simulink model "CDF_VSVPWM_Grid_Parallel"  (R2024b)
settings: Solver=VariableStepAuto  StartTime=0.0  StopTime=0.1  FixedStep=1e-7  MaxStep=1e-5  RelTol=1e-4
InitFcn:
  | Ts=1/10e3;
  | Udc=600;
  | C=4000e-6;
  | m=0.7;
libraries: simulink x24, spsMosfetLib x24, spsSeriesRLCBranchLib x16, spsDiodeLib x12, spsabctoAlphaBetaZeroLib x5, spsThreePhaseVIMeasurementLib x4, spsVoltageMeasurementLib x4, spsAlphaBetaZerotoabcLib x2, spsStairGeneratorLib x2, spsOnDelayLib x2, spsdq0toAlphaBetaZeroLib x2, spsBreakerLib x1
(part 1 of 5)
settings Model: SimulationMode=accelerator  PauseTimes=0  Creator=chenl  LastModifiedBy=ZKY
settings SolverCC: AlgebraicLoopSolver=TrustRegion
settings DataIOCC: ReturnWorkspaceOutputs=off
settings DebuggingCC: ArithmeticOperatorsInVariantConditions=warning
settings SFSimCC: CustomCodeUndefinedFunction=UseInterfaceOnly
settings RTWCC: GPUComputeCapability=3.5

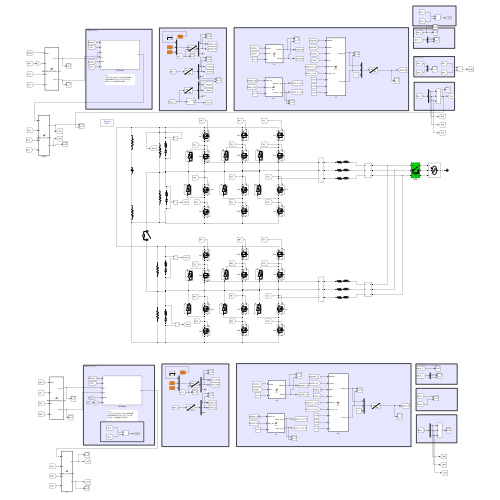
[thumbnail: Simulink's stored preview of the top-level diagram]
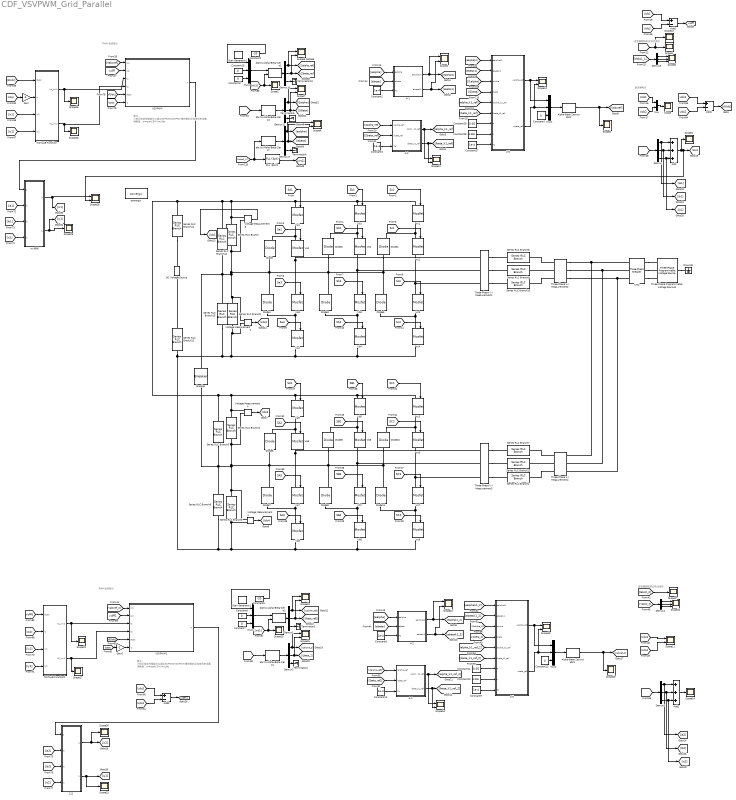
== system CDF_VSVPWM_Grid_Parallel ==
  note: PWM发波部分
  note: 备注：
      | 三相正弦信号幅值可以通过SVPWM/VSVPWM模块里的正弦信号来设置
      | 调制度：m=sqrt(3)*Vm/Udc
  note: 直流侧电压
  note: 逆变器侧线电压电流波形
  note: PWM发波部分
  note: 备注：
      | 三相正弦信号幅值可以通过SVPWM/VSVPWM模块里的正弦信号来设置
      | 调制度：m=sqrt(3)*Vm/Udc
  note: 逆变器侧线电压电流波形
  111 : MATLAB Function  TreatAsAtomicUnit=on
  4-1 : MATLAB Function  TreatAsAtomicUnit=on
  4-2 : MATLAB Function  TreatAsAtomicUnit=on
  4-3 : MATLAB Function  TreatAsAtomicUnit=on
  4-4 : MATLAB Function  TreatAsAtomicUnit=on
  4-5 : MATLAB Function  TreatAsAtomicUnit=on
  4-8 : MATLAB Function  TreatAsAtomicUnit=on
  Add : Sum  Inputs=+++
  Add1 : Sum  Inputs=+-
  Add2 : Sum  Inputs=+++
  Add3 : Sum  Inputs=+-
  Add4 : Sum  Inputs=+-
  Alpha-Beta-Zero to abc1 : spsAlphaBetaZerotoabcLib/Alpha-Beta-Zero to abc
      settings: LibrarySourceBlock=|
      | sps_lib/Control/Alpha-Beta-Zero
      | to abc
  Alpha-Beta-Zero to abc2 : spsAlphaBetaZerotoabcLib/Alpha-Beta-Zero to abc
      settings: LibrarySourceBlock=|
      | sps_lib/Control/Alpha-Beta-Zero
      | to abc
  Breaker : spsBreakerLib/Breaker  InitialState=0  SwitchingTimes=0  External=off  BreakerResistance=0.001  SnubberResistance=1e8  SnubberCapacitance=inf  Measurements=None  MoreParameters=on  NoBreakLoop=off
  Constant1 : Constant  Value=-20
  Constant10 : Constant  Value=0.005
  Constant11 : Constant  Value=0.05
  Constant{2..3,8..9,12,27} (6 alike) : Constant  Value=1e-4
  Constant{4,6..7,13,15,17} (6 alike) : Constant  Value=0
  Constant28 : Constant  Value=0.005
  Constant29 : Constant  Value=0.05
  Constant5 : Constant  Value=-20
  DC Voltage Source : spsDCVoltageSourceLib/DC Voltage Source  Amplitude=Udc  Measurements=None
  Demux{,1..5,10,12,17} (9 alike) : Demux  Outputs=3
  Diode{,1..11} (12 alike) : spsDiodeLib/Diode  Ron=0.001  Lon=0  Vf=0.8  IC=0  UseSnubber=0  Rs=500  Cs=250e-9  Measurements=on
  Gain : Gain  Gain=-1
  Gain2 : Gain  Gain=-1
  Ground3 : spsGroundLib/Ground  LConnTagsString=a
  KM4 : spsThreePhaseBreakerLib/Three-Phase Breaker  InitialState=open  SwitchA=on  SwitchB=on  SwitchC=on  SwitchTimes=0  External=off  BreakerResistance=0.001  SnubberResistance=1e6  SnubberCapacitance=inf  Measurements=None
  MATLAB Function : MATLAB Function  TreatAsAtomicUnit=on
  MATLAB Function1 : MATLAB Function  TreatAsAtomicUnit=on
  Mux : Mux  Inputs=2
  Mux{1..4} (4 alike) : Mux  Inputs=3
  PLL (3ph)2 : spsPLL3phLib/PLL (3ph)  Fmin=45  Par_Init=[0, 50]  ParK=[180, 3200, 0]  TcD=1e-4  MaxRateChangeFreq=12  FilterCutOffFreq=25  Ts=0  AGC=on
  Scope{,1..3,8,10,14..17,20..21,26..27,29..31} (17 alike) : Scope
  Scope{4..5,7,9,11..13,18..19,22..23,25,28} (13 alike) : Scope  NumInputPorts=2
  Scope24 : Scope  NumInputPorts=3
  Scope6 : Scope  NumInputPorts=3
  Series RLC Branch : spsSeriesRLCBranchLib/Series RLC Branch  LConnTagsString=__new0  RConnTagsString=__new0  BranchType=RC  Resistance=1  Inductance=1e-3  SetiL0=off  InitialCurrent=0  Capacitance=860e-6  Setx0=on  InitialVoltage=280  Measurements=None
  Series RLC Branch1 : spsSeriesRLCBranchLib/Series RLC Branch  LConnTagsString=__new0  RConnTagsString=__new0  BranchType=RC  Resistance=0.001  Inductance=1e-3  SetiL0=off  InitialCurrent=0  Capacitance=860e-6  Setx0=on  InitialVoltage=320  Measurements=None
  Series RLC Branch10 : spsSeriesRLCBranchLib/Series RLC Branch  LConnTagsString=__new0  RConnTagsString=__new0  BranchType=RL  Resistance=0.05  Inductance=3e-3  SetiL0=off  InitialCurrent=0  Capacitance=1e-6  Setx0=off  InitialVoltage=0  Measurements=None
  Series RLC Branch11 : spsSeriesRLCBranchLib/Series RLC Branch  LConnTagsString=__new0  RConnTagsString=__new0  BranchType=R  Resistance=0.00001  Inductance=1e-3  SetiL0=off  InitialCurrent=0  Capacitance=1e-6  Setx0=off  InitialVoltage=0  Measurements=None
  Series RLC Branch12 : spsSeriesRLCBranchLib/Series RLC Branch  LConnTagsString=__new0  RConnTagsString=__new0  BranchType=R  Resistance=0.00001  Inductance=1e-3  SetiL0=off  InitialCurrent=0  Capacitance=1e-6  Setx0=off  InitialVoltage=0  Measurements=None
  Series RLC Branch13 : spsSeriesRLCBranchLib/Series RLC Branch  LConnTagsString=__new0  RConnTagsString=__new0  BranchType=RL  Resistance=0.05  Inductance=5e-3  SetiL0=off  InitialCurrent=0  Capacitance=1e-6  Setx0=off  InitialVoltage=0  Measurements=None
  Series RLC Branch{5..6,14..15} (4 alike) : spsSeriesRLCBranchLib/Series RLC Branch  LConnTagsString=__new0  RConnTagsString=__new0  BranchType=R  Resistance=1e4  Inductance=1e-3  SetiL0=off  InitialCurrent=0  Capacitance=C  Setx0=on  InitialVoltage=Udc/2  Measurements=None
  Series RLC Branch2 : spsSeriesRLCBranchLib/Series RLC Branch  LConnTagsString=__new0  RConnTagsString=__new0  BranchType=RC  Resistance=0.001  Inductance=1e-3  SetiL0=off  InitialCurrent=0  Capacitance=860e-6  Setx0=off  InitialVoltage=300  Measurements=None
  Series RLC Branch3 : spsSeriesRLCBranchLib/Series RLC Branch  LConnTagsString=__new0  RConnTagsString=__new0  BranchType=RC  Resistance=0.001  Inductance=1e-3  SetiL0=off  InitialCurrent=0  Capacitance=860e-6  Setx0=off  InitialVoltage=300  Measurements=None
  Series RLC Branch4 : spsSeriesRLCBranchLib/Series RLC Branch  LConnTagsString=__new0  RConnTagsString=__new0  BranchType=RL  Resistance=0.05  Inductance=5e-3  SetiL0=off  InitialCurrent=0  Capacitance=1e-6  Setx0=off  InitialVoltage=0  Measurements=None
  Series RLC Branch7 : spsSeriesRLCBranchLib/Series RLC Branch  LConnTagsString=__new0  RConnTagsString=__new0  BranchType=RL  Resistance=0.05  Inductance=5e-3  SetiL0=off  InitialCurrent=0  Capacitance=1e-6  Setx0=off  InitialVoltage=0  Measurements=None
  Series RLC Branch8 : spsSeriesRLCBranchLib/Series RLC Branch  LConnTagsString=__new0  RConnTagsString=__new0  BranchType=RL  Resistance=0.05  Inductance=3e-3  SetiL0=off  InitialCurrent=0  Capacitance=1e-6  Setx0=off  InitialVoltage=0  Measurements=None
  Series RLC Branch9 : spsSeriesRLCBranchLib/Series RLC Branch  LConnTagsString=__new0  RConnTagsString=__new0  BranchType=RL  Resistance=0.05  Inductance=3e-3  SetiL0=off  InitialCurrent=0  Capacitance=1e-6  Setx0=off  InitialVoltage=0  Measurements=None
  Stair Generator1 : spsStairGeneratorLib/Stair Generator  t=[0, 0.1, 0.2]  e=[-10,-5, -10]  Ts=0
  Stair Generator2 : spsStairGeneratorLib/Stair Generator  t=[0, 0.1, 0.2]  e=[-10,-5, -10]  Ts=0
  Terminator{1..2,6,8,10} (5 alike) : Terminator
  Three-Phase Programmable Voltage Source1 : spsThreePhaseProgrammableVoltageSourceLib/Three-Phase Programmable Voltage Source  PositiveSequence="[380  0 50]"  VariationEntity=Amplitude  VariationType=Table of time-amplitude pairs  VariationTypeAlt=Step  VariationStep=0.5  VariationRate=10  VariationMagnitude=0.3  VariationFrequency=2  VariationTiming=[1 2]  VariationPhaseA=on  Amplitudes=[1.0]  TimeValues=[0]  HarmonicGeneration=off  HarmonicA=[3 0.064 0 0]  HarmonicB=[5 0.048 0 2]  Timing="[0.05  0.15]"  BusType=swing  Pref=0  Qref=0  Qmin=-inf  Qmax=inf
  Three-Phase V-I Measurement1 : spsThreePhaseVIMeasurementLib/Three-Phase V-I Measurement  VoltageMeasurement=phase-to-ground  SetLabelV=on  LabelV=Vabc1_1  Vpu=off  VpuLL=off  CurrentMeasurement=yes  SetLabelI=on  LabelI=Iabc1_1  Ipu=off  Pbase=100e6  Vbase=500e3  OutputType=Complex  PhasorSimulation=off  PSBequivalent=0
  Three-Phase V-I Measurement2 : spsThreePhaseVIMeasurementLib/Three-Phase V-I Measurement  VoltageMeasurement=phase-to-ground  SetLabelV=on  LabelV=Vabc2_1  Vpu=off  VpuLL=off  CurrentMeasurement=no  SetLabelI=on  LabelI=Iabc2  Ipu=off  Pbase=100e6  Vbase=500e3  OutputType=Complex  PhasorSimulation=off  PSBequivalent=0
  Three-Phase V-I Measurement3 : spsThreePhaseVIMeasurementLib/Three-Phase V-I Measurement  VoltageMeasurement=phase-to-ground  SetLabelV=on  LabelV=Vabc1_2  Vpu=off  VpuLL=off  CurrentMeasurement=yes  SetLabelI=on  LabelI=Iabc1_2  Ipu=off  Pbase=100e6  Vbase=500e3  OutputType=Complex  PhasorSimulation=off  PSBequivalent=0
  Three-Phase V-I Measurement4 : spsThreePhaseVIMeasurementLib/Three-Phase V-I Measurement  VoltageMeasurement=phase-to-ground  SetLabelV=on  LabelV=Vabc2_2  Vpu=off  VpuLL=off  CurrentMeasurement=yes  SetLabelI=on  LabelI=Iabc2_2  Ipu=off  Pbase=100e6  Vbase=500e3  OutputType=Complex  PhasorSimulation=off  PSBequivalent=0
  VSVPWM : Subsystem
  VSVPWM1 : Subsystem
  Va{1..8} (8 alike) : spsMosfetLib/Mosfet  Ron=0.1  Lon=0  Rd=0.01  Vfd=0  IC=0  Rs=1e5  Cs=inf  Measurements=on
  Vb{1..8} (8 alike) : spsMosfetLib/Mosfet  Ron=0.1  Lon=0  Rd=0.01  Vfd=0  IC=0  Rs=1e5  Cs=inf  Measurements=on
  Vc{1..8} (8 alike) : spsMosfetLib/Mosfet  Ron=0.1  Lon=0  Rd=0.01  Vfd=0  IC=0  Rs=1e5  Cs=inf  Measurements=on
  Voltage Measurement{1..2,4,8} (4 alike) : spsVoltageMeasurementLib/Voltage Measurement  PhasorSimulation=off  OutputType=Complex  PSBequivalent=0
  abc to Alpha-Beta-Zero1 : spsabctoAlphaBetaZeroLib/abc to Alpha-Beta-Zero
  abc to Alpha-Beta-Zero4 : spsabctoAlphaBetaZeroLib/abc to Alpha-Beta-Zero
  abc to Alpha-Beta-Zero7 : spsabctoAlphaBetaZeroLib/abc to Alpha-Beta-Zero
  dq0 to Alpha-Beta-Zero1 : spsdq0toAlphaBetaZeroLib/dq0 to Alpha-Beta-Zero  Alignment=90 degrees behind phase A axis
  dq0 to Alpha-Beta-Zero2 : spsdq0toAlphaBetaZeroLib/dq0 to Alpha-Beta-Zero  Alignment=90 degrees behind phase A axis
  ixiy映射 : MATLAB Function  TreatAsAtomicUnit=on
  powergui : spspowerguiLib/powergui  Priority=1  SimulationMode=Discrete  SampleTime=1e-6  frequency=60  Iterations=50  frequencyindice=0  Pbase=100e6  ErrMax=1e-4  UnitsV=kV  UnitsW=MW  buscounter=0  FunctionMessages=off  echomessages=off  EnableUseOfTLC=off  CurrentSourceSwitches=off  DisableSnubberDevices=off  DisableRonSwitches=off  DisableVfSwitches=off  DisplayEquations=off  AutomaticDiscreteSolvers=off  SolverType=Tustin/Backward Euler (TBE)  Interpol=off  ExternalGateDelay=off  methode=off  Ts=100  x0status=blocks  SPID=on  SwTol=0  HookPort=off  NonlinearTolerance=1e-4  nMaxIteration=100  ContinueOnMaxIteration=off  RestoreLinks=warning  ResistiveCurrentMeasurement=off  Frange=[0:2:500]  Ylog=off  Xlog=on  ShowGrid=off  save=off  variable=ZData  structure=0  ZoomFFT=off  StartTime=0.0  cycles=1  DisplayStyle=1  fundamental=60  FreqAxis=off  MaxFrequency=1000  frequencyindicesteady=1  RmsSteady=1  display=off
  tag scope: Udc1=global, Udc2=global, Udc3=global, Udc4=global, izscc=global, vidff1=global, vidff=global
  wires:
  [Sa1] -> Va1/1
  [Sb1] -> Vb1/1
  [Sc1] -> Vc1/1
  [Sa4] -> Va4/1
  [Sb4] -> Vb4/1
  [Sc4] -> Vc4/1
  [Iabc1_1] -> Scope10/1, Scope1/1
  Voltage Measurement2/1 -> [Udc2]
  [Vabc1_1] -> Demux5/1
  Demux5/1 -> Scope6/1
  Demux5/2 -> Scope6/2
  Demux5/3 -> Scope6/3
  Voltage Measurement8/1 -> [Udc1]
  [Sa2] -> Va2/1
  [Sb2] -> Vb2/1
  [Sc2] -> Vc2/1
  [Sa3] -> Va3/1
  [Sb3] -> Vb3/1
  [Sc3] -> Vc3/1
  Mux4/1 -> dq0 to Alpha-Beta-Zero1/1
  Demux10/1 -> Scope5/1, [i2alpha_ref]
  Demux10/3 -> Terminator10/1
  Constant17/1 -> Mux4/3
  Demux10/2 -> Scope5/2, [i2beta_ref]
  [wt1] -> Scope2/1, dq0 to Alpha-Beta-Zero1/2
  Constant15/1 -> Mux4/2
  dq0 to Alpha-Beta-Zero1/1 -> Demux10/1
  [Iabc1_1] -> abc to Alpha-Beta-Zero4/1
  Demux12/3 -> Terminator6/1
  Demux12/2 -> Scope7/2, [I1beta]
  Demux12/1 -> Scope7/1, [I1alpha]
  abc to Alpha-Beta-Zero4/1 -> Demux12/1
  Demux17/2 -> Scope9/2, [ebeta]
  Demux17/3 -> Terminator8/1
  Demux17/1 -> Scope9/1, [ealpha]
  [Vabc2_1] -> abc to Alpha-Beta-Zero7/1, PLL (3ph)2/1
  PLL (3ph)2/2 -> [wt1]
  abc to Alpha-Beta-Zero7/1 -> Demux17/1
  Constant2/1 -> 4-1/3(Ts)
  [ealpha] -> 4-1/1(ealpha)
  [ebeta] -> 4-1/2(ebeta)
  4-1/2(ebetak1) -> [ebetak1], Scope4/2
  4-1/1(ealphak1) -> Scope4/1, [ealphak1]
  [i2alpha_ref] -> 4-3/1(ialpha_ref)
  [i2beta_ref] -> 4-3/2(ibeta_ref)
  Constant3/1 -> 4-3/3(Ts)
  [ealphak1] -> 4-8/1(ealphak1)
  [ebetak1] -> 4-8/2(ebetak1)
  [I1alpha] -> 4-8/3(ialpha)
  [I1beta] -> 4-8/4(ibeta)
  4-3/1(ialpha_k1_ref) -> Scope12/1, [ialpha_k1_ref]
  4-3/2(ibeta_k1_ref) -> Scope12/2, [ibeta_k1_ref]
  [ialpha_k1_ref] -> 4-8/5(ialpha_k1_ref)
  [ibeta_k1_ref] -> 4-8/6(ibeta_k1_ref)
  Constant29/1 -> 4-8/7(R)
  Constant28/1 -> 4-8/8(L)
  Constant27/1 -> 4-8/9(Ts)
  Constant4/1 -> Mux1/3
  Alpha-Beta-Zero to abc2/1 -> Scope8/1, [Vabcref]
  4-8/1(ualpha_ref) -> Scope13/1, Mux1/1
  4-8/2(ubeta_ref) -> Scope13/2, Mux1/2
  Mux1/1 -> Alpha-Beta-Zero to abc2/1
  [Vabcref] -> VSVPWM/1(In1)
  Constant1/1 -> Mux4/1
  [SA1] -> Va5/1
  Voltage Measurement1/1 -> [Udc4]
  [SA3] -> Va7/1
  Voltage Measurement4/1 -> [Udc3]
  [SC2] -> Vc6/1
  [SB4] -> Vb8/1
  [SC1] -> Vc5/1
  [SB1] -> Vb5/1
  [SB2] -> Vb6/1
  [SB3] -> Vb7/1
  [SC4] -> Vc8/1
  [SA4] -> Va8/1
  [SA2] -> Va6/1
  [SC3] -> Vc7/1
  Alpha-Beta-Zero to abc1/1 -> Scope26/1, [Vabcref_2]
  [ealpha] -> 4-2/1(ealpha)
  [i2alpha_ref2] -> 4-4/1(ialpha_ref)
  Constant6/1 -> Mux3/2
  Demux4/1 -> Scope24/1
  [Iabc2_2] -> Scope17/1
  [ibeta_k1_ref_2] -> 4-5/6(ibeta_k1_ref)
  Mux2/1 -> Alpha-Beta-Zero to abc1/1
  [ialpha_k1_ref_2] -> 4-5/5(ialpha_k1_ref)
  Constant7/1 -> Mux3/3
  [Vabc2_2] -> Demux4/1
  Demux1/2 -> Scope23/2, [i2beta_ref2]
  Demux1/1 -> Scope23/1, [i2alpha_ref2]
  abc to Alpha-Beta-Zero1/1 -> Demux2/1
  dq0 to Alpha-Beta-Zero2/1 -> Demux1/1
  [ebetak1_2] -> 4-5/2(ebetak1)
  [Iabc1_2] -> abc to Alpha-Beta-Zero1/1
  [Vabcref_2] -> VSVPWM1/1(In1)
  [i2beta_ref2] -> 4-4/2(ibeta_ref)
  Constant5/1 -> Mux3/1
  4-2/1(ealphak1) -> Scope22/1, [ealphak1_2]
  Demux1/3 -> Terminator1/1
  Demux2/2 -> Scope25/2, [I1beta_2]
  [I1beta_2] -> 4-5/4(ibeta)
  Constant12/1 -> 4-4/3(Ts)
  Constant10/1 -> 4-5/8(L)
  Demux4/3 -> Scope24/3
  Mux3/1 -> dq0 to Alpha-Beta-Zero2/1
  Demux2/1 -> Scope25/1, [I1alpha_2]
  [ealphak1_2] -> 4-5/1(ealphak1)
  4-5/2(ubeta_ref) -> Scope19/2, Mux2/2
  [wt1] -> Scope20/1, dq0 to Alpha-Beta-Zero2/2
  4-2/2(ebetak1) -> [ebetak1_2], Scope22/2
  Constant8/1 -> 4-2/3(Ts)
  4-5/1(ualpha_ref) -> Scope19/1, Mux2/1
  Demux2/3 -> Terminator2/1
  Constant13/1 -> Mux2/3
  4-4/1(ialpha_k1_ref) -> Scope18/1, [ialpha_k1_ref_2]
  4-4/2(ibeta_k1_ref) -> Scope18/2, [ibeta_k1_ref_2]
  Demux4/2 -> Scope24/2
  [I1alpha_2] -> 4-5/3(ialpha)
  [ebeta] -> 4-2/2(ebeta)
  Constant9/1 -> 4-5/9(Ts)
  Constant11/1 -> 4-5/7(R)
  [Iabc1_1] -> Demux/1
  Demux/1 -> Add/1, [ia1]
  Demux/2 -> Add/2, [ib1]
  Demux/3 -> [ic1], Add/3
  Add/1 -> Scope3/1, Scope28/2, [izscc]
  [vidff] -> VSVPWM/2(In2)
  [Udc2] -> Add1/2
  [Udc1] -> Add1/1
  Add1/1 -> [vidff]
  [Udc3] -> Scope11/1
  [Udc4] -> Scope11/2
  Demux3/3 -> [ic2], Add2/3
  [Iabc1_1] -> Demux3/1
  Add2/1 -> Scope14/1
  Demux3/1 -> [ia2], Add2/1
  [vidff1] -> VSVPWM1/2(In2)
  Add3/1 -> [vidff1]
  [Udc4] -> Add3/2
  [Udc3] -> Add3/1
  [Udc1] -> Mux/1
  [Udc2] -> Mux/2
  Mux/1 -> Scope/1
  [Udc1] -> Add4/1
  [Udc2] -> Add4/2
  Add4/1 -> [dUdc]
  [dUdc] -> MATLAB Function/1(dUdc)
  [ix1] -> MATLAB Function/3(ix1)
  [iy1] -> MATLAB Function/4(iy1)
  MATLAB Function/1(k1_inv1) -> Scope15/1, VSVPWM/3(k1)
  MATLAB Function/2(k2_inv1) -> VSVPWM/4(k2), Scope16/1
  [izscc] -> Gain/1
  Gain/1 -> MATLAB Function/2(iz)
  [ix2] -> MATLAB Function1/3(ix1)
  [iy2] -> MATLAB Function1/4(iy1)
  [vidff1] -> MATLAB Function1/1(dUdc)
  Demux3/2 -> [ib2], Add2/2
  MATLAB Function1/1(k1_inv1) -> Scope21/1, VSVPWM1/3(k1)
  MATLAB Function1/2(k2_inv1) -> Scope27/1, VSVPWM1/4(k2)
  VSVPWM/1(N) -> ixiy映射/1(N)
  [ia1] -> ixiy映射/2(ia)
  [ic1] -> ixiy映射/4(ic)
  [ib1] -> ixiy映射/3(ib)
  ixiy映射/1(ix) -> [ix1], Scope28/1
  ixiy映射/2(iy) -> [iy1], Scope29/1
  111/2(iy) -> [iy2], Scope31/1
  VSVPWM1/1(N) -> 111/1(N)
  [ia2] -> 111/2(ia)
  111/1(ix) -> [ix2], Scope30/1
  [ic2] -> 111/4(ic)
  [ib2] -> 111/3(ib)
  [dUdc] -> VSVPWM/5(dUdc)
  [izscc] -> VSVPWM/6(iz)
  [dUdc] -> VSVPWM1/5(dUdc)
  Gain2/1 -> VSVPWM1/6(iz)
  [izscc] -> Gain2/1
  [izscc] -> MATLAB Function1/2(iz)
  connections:
  Series RLC Branch3/RConn1 -- Vb8/RConn1 -- Vc8/RConn1 -- Va8/RConn1 -- Series RLC Branch15/RConn1 -- Series RLC Branch1/RConn1 -- Voltage Measurement2/LConn2 -- Vc4/RConn1 -- Vb4/RConn1 -- Va4/RConn1 -- Series RLC Branch12/RConn1 -- Series RLC Branch6/RConn1 -- Voltage Measurement1/LConn2
  Three-Phase V-I Measurement1/RConn3 -- Series RLC Branch10/LConn1
  Three-Phase V-I Measurement1/RConn2 -- Series RLC Branch9/LConn1
  Three-Phase V-I Measurement1/RConn1 -- Series RLC Branch8/LConn1
  Series RLC Branch11/LConn1 -- DC Voltage Source/RConn1
  Series RLC Branch12/LConn1 -- DC Voltage Source/LConn1
  Va2/LConn1 -- Va1/RConn1 -- Diode/RConn1
  Vb2/LConn1 -- Diode2/RConn1 -- Vb1/RConn1
  Vc2/LConn1 -- Diode4/RConn1 -- Vc1/RConn1
  Va2/RConn1 -- Va3/LConn1 -- Three-Phase V-I Measurement1/LConn1
  Va3/RConn1 -- Diode1/LConn1 -- Va4/LConn1
  Vb3/RConn1 -- Diode3/LConn1 -- Vb4/LConn1
  Vb3/LConn1 -- Vb2/RConn1 -- Three-Phase V-I Measurement1/LConn2
  Vc3/RConn1 -- Vc4/LConn1 -- Diode5/LConn1
  Vc3/LConn1 -- Three-Phase V-I Measurement1/LConn3 -- Vc2/RConn1
  Three-Phase V-I Measurement2/RConn3 -- Series RLC Branch10/RConn1
  Three-Phase Programmable Voltage Source1/LConn1 -- Ground3/LConn1
  Three-Phase V-I Measurement2/RConn2 -- Series RLC Branch9/RConn1
  Three-Phase V-I Measurement2/RConn1 -- Series RLC Branch8/RConn1
  Va8/LConn1 -- Va7/RConn1 -- Diode7/LConn1
  Vc6/RConn1 -- Vc7/LConn1 -- Three-Phase V-I Measurement3/LConn3
  Three-Phase V-I Measurement3/LConn1 -- Va6/RConn1 -- Va7/LConn1
  Diode11/LConn1 -- Vc7/RConn1 -- Vc8/LConn1
  Diode6/RConn1 -- Va6/LConn1 -- Va5/RConn1
  Vb5/RConn1 -- Vb6/LConn1 -- Diode8/RConn1
  Vb8/LConn1 -- Vb7/RConn1 -- Diode9/LConn1
  Vc5/RConn1 -- Vc6/LConn1 -- Diode10/RConn1
  Three-Phase V-I Measurement3/LConn2 -- Vb7/LConn1 -- Vb6/RConn1
  Three-Phase V-I Measurement3/RConn3 -- Series RLC Branch4/LConn1
  Three-Phase V-I Measurement4/RConn2 -- Series RLC Branch13/RConn1
  Three-Phase V-I Measurement3/RConn2 -- Series RLC Branch13/LConn1
  Three-Phase V-I Measurement4/RConn3 -- Series RLC Branch4/RConn1
  Three-Phase V-I Measurement4/RConn1 -- Series RLC Branch7/RConn1
  Three-Phase V-I Measurement3/RConn1 -- Series RLC Branch7/LConn1
  Three-Phase V-I Measurement2/LConn1 -- KM4/LConn1 -- Three-Phase V-I Measurement4/LConn1
  Three-Phase V-I Measurement2/LConn2 -- KM4/LConn2 -- Three-Phase V-I Measurement4/LConn2
  Three-Phase V-I Measurement2/LConn3 -- KM4/LConn3 -- Three-Phase V-I Measurement4/LConn3
  Series RLC Branch6/LConn1 -- Series RLC Branch5/RConn1 -- Breaker/RConn1 -- Series RLC Branch3/LConn1 -- Voltage Measurement1/LConn1 -- Voltage Measurement4/LConn2 -- Series RLC Branch2/RConn1 -- Diode7/RConn1 -- Diode9/RConn1 -- Diode11/RConn1 -- Diode10/LConn1 -- Diode8/LConn1 -- Diode6/LConn1
  Vb5/LConn1 -- Series RLC Branch2/LConn1 -- Voltage Measurement4/LConn1 -- Series RLC Branch5/LConn1 -- Series RLC Branch14/LConn1 -- Series RLC Branch/LConn1 -- Voltage Measurement8/LConn1 -- Vc1/LConn1 -- Vb1/LConn1 -- Va1/LConn1 -- Series RLC Branch11/RConn1 -- Va5/LConn1 -- Vc5/LConn1
  Series RLC Branch1/LConn1 -- Breaker/LConn1 -- Series RLC Branch15/LConn1 -- Series RLC Branch14/RConn1 -- Diode3/RConn1 -- Diode5/RConn1 -- Diode4/LConn1 -- Diode2/LConn1 -- Diode/LConn1 -- Diode1/RConn1 -- Voltage Measurement8/LConn2 -- Series RLC Branch/RConn1 -- Voltage Measurement2/LConn1
  KM4/RConn1 -- Three-Phase Programmable Voltage Source1/RConn1
  KM4/RConn2 -- Three-Phase Programmable Voltage Source1/RConn2
  KM4/RConn3 -- Three-Phase Programmable Voltage Source1/RConn3

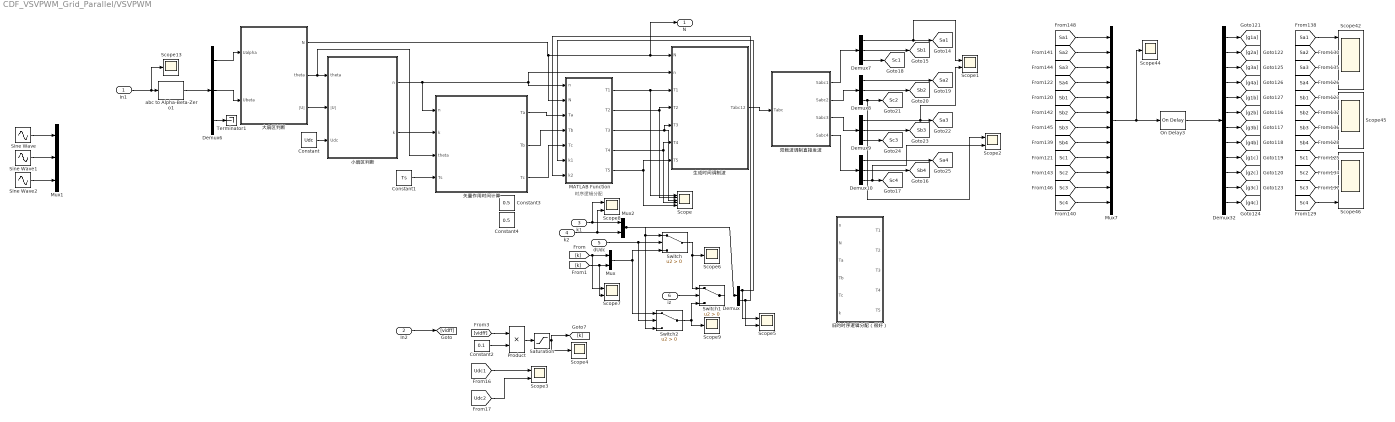
== system CDF_VSVPWM_Grid_Parallel/VSVPWM ==
  note: 时序逻辑分配
  In1 : Inport
  In2 : Inport  Port=2
  k1 : Inport  Port=3
  k2 : Inport  Port=4
  dUdc : Inport  Port=5
  iz : Inport  Port=6
  Constant : Constant  Value=Udc
  Constant1 : Constant  Value=Ts
  Constant2 : Constant  Value=0.1
  Constant3 : Constant  Value=0.5
  Constant4 : Constant  Value=0.5
  Demux : Demux  Outputs=2
  Demux{6..10} (5 alike) : Demux  Outputs=3
  Demux32 : Demux  Outputs=12
  MATLAB Function : MATLAB Function  TreatAsAtomicUnit=on
  Mux : Mux  Inputs=2
  Mux1 : Mux  Inputs=3
  Mux2 : Mux  Inputs=2
  Mux7 : Mux  Inputs=12
  On Delay3 : spsOnDelayLib/On Delay  DelayType=On delay  delay=250e-9  ic=0  Ts=0
  Product : Product
  Saturation : Saturate  UpperLimit=1  LowerLimit=-1
  Scope : Scope  NumInputPorts=5
  Scope{1..3,5,7..8} (6 alike) : Scope  NumInputPorts=2
  Scope{4,6,9,13,44} (5 alike) : Scope
  Scope42 : Scope  NumInputPorts=4
  Scope45 : Scope  NumInputPorts=4
  Scope46 : Scope  NumInputPorts=4
  Sine Wave : Sin  Amplitude=m*Udc/sqrt(3)  Frequency=100*pi  SampleTime=0
  Sine Wave1 : Sin  Amplitude=m*Udc/sqrt(3)  Frequency=100*pi  Phase=-2*pi/3  SampleTime=0
  Sine Wave2 : Sin  Amplitude=m*Udc/sqrt(3)  Frequency=100*pi  Phase=2*pi/3  SampleTime=0
  Switch : Switch  Criteria=u2 > Threshold  SaturateOnIntegerOverflow=off  InputSameDT=off
  Switch1 : Switch  Criteria=u2 > Threshold  SaturateOnIntegerOverflow=off  InputSameDT=off
  Switch2 : Switch  Criteria=u2 > Threshold  SaturateOnIntegerOverflow=off  InputSameDT=off
  Terminator1 : Terminator
  abc to Alpha-Beta-Zero1 : spsabctoAlphaBetaZeroLib/abc to Alpha-Beta-Zero
  双载波调制直接发波 : Subsystem
  大扇区判断 : Subsystem
  小扇区判断 : Subsystem
  旧的时序逻辑分配（很好） : MATLAB Function  TreatAsAtomicUnit=on
  生成时间调制波 : Subsystem
  矢量作用时间计算 : Subsystem
  N : Outport
  tag scope: Sa1=global, Sa2=global, Sa3=global, Sa4=global, Sb1=global, Sb2=global, Sb3=global, Sb4=global, Sc1=global, Sc2=global, Sc3=global, Sc4=global
  wires:
  大扇区判断/2(theta) -> 矢量作用时间计算/3(theta), 小扇区判断/1(theta)
  大扇区判断/3(|U|) -> 小扇区判断/2(|U|)
  Constant/1 -> 小扇区判断/3(Udc)
  Demux6/2 -> 大扇区判断/2(Ubeta)
  abc to Alpha-Beta-Zero1/1 -> Demux6/1
  In1/1 -> Scope13/1, abc to Alpha-Beta-Zero1/1
  Demux6/1 -> 大扇区判断/1(Ualpha)
  小扇区判断/2(k) -> 矢量作用时间计算/2(k)
  小扇区判断/1(n) -> 矢量作用时间计算/1(n), MATLAB Function/1(n), 生成时间调制波/2(n)
  Constant1/1 -> 矢量作用时间计算/4(Ts)
  大扇区判断/1(N) -> MATLAB Function/2(N), N/1, 生成时间调制波/1(N)
  生成时间调制波/1(Tabc12) -> 双载波调制直接发波/1(Tabc)
  Demux9/3 -> [Sc3]
  Demux9/2 -> [Sb3]
  Demux8/1 -> [Sa2]
  Demux10/2 -> [Sb4]
  Demux10/3 -> Scope2/2, [Sc4]
  Demux7/1 -> Scope1/1, [Sa1]
  Demux10/1 -> [Sa4]
  Demux8/2 -> [Sb2]
  Demux7/2 -> [Sb1]
  Demux7/3 -> [Sc1]
  Demux9/1 -> Scope1/2, [Sa3]
  Demux8/3 -> Scope2/1, [Sc2]
  双载波调制直接发波/1(Sabc1) -> Demux7/1
  双载波调制直接发波/2(Sabc2) -> Demux8/1
  双载波调制直接发波/3(Sabc3) -> Demux9/1
  双载波调制直接发波/4(Sabc4) -> Demux10/1
  Demux6/3 -> Terminator1/1
  MATLAB Function/1(T1) -> Scope/1, 生成时间调制波/3(T1)
  MATLAB Function/2(T2) -> Scope/2, 生成时间调制波/4(T2)
  MATLAB Function/3(T3) -> Scope/3, 生成时间调制波/5(T3)
  MATLAB Function/4(T4) -> Scope/4, 生成时间调制波/6(T4)
  MATLAB Function/5(T5) -> Scope/5, 生成时间调制波/7(T5)
  [Sc3] -> Scope46/3
  [Sa3] -> Scope42/3
  Demux32/11 -> [g3c]
  Demux32/2 -> [g2a]
  On Delay3/1 -> Demux32/1
  Demux32/5 -> [g1b]
  Demux32/12 -> [g4c]
  Demux32/1 -> [g1a]
  Demux32/10 -> [g2c]
  Demux32/7 -> [g3b]
  Demux32/8 -> [g4b]
  Demux32/6 -> [g2b]
  [Sb3] -> Mux7/7
  Mux7/1 -> On Delay3/1, Scope44/1
  [Sc1] -> Mux7/9
  [Sb1] -> Scope45/1
  [Sb2] -> Scope45/2
  [Sa2] -> Scope42/2
  [Sb3] -> Scope45/3
  Demux32/9 -> [g1c]
  [Sb4] -> Scope45/4
  Demux32/3 -> [g3a]
  [Sb1] -> Mux7/5
  [Sa1] -> Mux7/1
  [Sa3] -> Mux7/3
  [Sb2] -> Mux7/6
  [Sa2] -> Mux7/2
  [Sb4] -> Mux7/8
  Demux32/4 -> [g4a]
  [Sa4] -> Mux7/4
  [Sc4] -> Mux7/12
  [Sc4] -> Scope46/4
  [Sc2] -> Scope46/2
  [Sa1] -> Scope42/1
  [Sc3] -> Mux7/11
  [Sc2] -> Mux7/10
  [Sa4] -> Scope42/4
  [Sc1] -> Scope46/1
  Sine Wave1/1 -> Mux1/2
  Sine Wave2/1 -> Mux1/3
  Sine Wave/1 -> Mux1/1
  In2/1 -> [vidff]
  Constant2/1 -> Product/2
  [vidff] -> Product/1
  [Udc2] -> Scope3/2
  Product/1 -> Saturation/1
  Saturation/1 -> [k], Scope4/1
  [Udc1] -> Scope3/1
  矢量作用时间计算/1(Ta) -> MATLAB Function/3(Ta)
  矢量作用时间计算/2(Tb) -> MATLAB Function/4(Tb)
  矢量作用时间计算/3(Tc) -> MATLAB Function/5(Tc)
  k1/1 -> Scope8/1, Mux2/1
  k2/1 -> Scope8/2, Mux2/2
  dUdc/1 -> Switch/2, Switch2/2
  iz/1 -> Switch1/2
  [k] -> Scope7/1, Mux/1
  [k] -> Scope7/2, Mux/2
  Switch/1 -> Scope6/1, Switch1/1
  Mux2/1 -> Switch2/3, Switch/1, Demux/1
  Mux/1 -> Switch2/1, Switch/3
  Switch2/1 -> Switch1/3, Scope9/1
  Demux/1 -> Scope5/1, MATLAB Function/6(k1)
  Demux/2 -> Scope5/2, MATLAB Function/7(k2)
